simulink model "TosAeb"  (R2021a) (continued)
== system TosAeb/ELK_Objsel/Select Object Info2/If Action Subsystem1 ==
  Index : Inport
  Objs_all : Inport  Port=2
  Action Port : ActionPort  ActionPortLabel=else
  Selector : Selector  InputPortWidth=32  IndexOptions=Index vector (port)  Indices=[1 3]  OutputSizes=1
  TargetInfo : Outport
  wires:
  Selector/1 -> TargetInfo/1
  [Index] -> Selector/2
  [Objs_all] -> Selector/1
  Objs_all/1 -> [Objs_all]
  Index/1 -> [Index]

== system TosAeb/ELK_Objsel/Select Object Info2/If Action Subsystem2 ==
  Action Port : ActionPort  ActionPortLabel=if(u1 == 255)
  Bus Creator1 : BusCreator  Inputs=64  OutDataTypeStr=Bus: Object_Struct  NonVirtualBus=on
  Constant : Constant  Value=0  OutDataTypeStr=int64_t
  Constant{1..2,4,8,14..18,20..22,24..27,29..36,38..42,47,50..55,57,62,64..72,74} (48 alike) : Constant  Value=0  OutDataTypeStr=single
  Constant10 : Constant  Value=0  OutDataTypeStr=uint16_t
  Constant11 : Constant  Value=0  OutDataTypeStr=uint16_t
  Constant12 : Constant  Value=0  OutDataTypeStr=bool_t
  Constant{6..7,9,13,19,37,63,73} (8 alike) : Constant  Value=0  OutDataTypeStr=uint8_t
  Constant23 : Constant  Value=100  OutDataTypeStr=single
  Constant28 : Constant  Value=0  OutDataTypeStr=bool_t
  Constant3 : Constant  Value=0  OutDataTypeStr=uint16_t
  Constant5 : Constant  Value=0  OutDataTypeStr=int64_t
  Out : Outport  OutDataTypeStr=Bus:Object_Struct
  wires:
  Constant47/1 -> Bus Creator1/45  "objLengthStd"
  Constant42/1 -> Bus Creator1/44  "objHeight"
  Constant41/1 -> Bus Creator1/42  "objLength"
  Constant40/1 -> Bus Creator1/43  "objWidth"
  Constant39/1 -> Bus Creator1/41  "yawRateStd"
  Constant38/1 -> Bus Creator1/40  "yawRate"
  Constant31/1 -> Bus Creator1/35  "ayAbsStd"
  Constant33/1 -> Bus Creator1/33  "vyAbsStd"
  Constant34/1 -> Bus Creator1/34  "axAbsStd"
  Constant53/1 -> Bus Creator1/36  "yawAngle"
  Constant55/1 -> Bus Creator1/38  "azimuthAngle"
  Constant54/1 -> Bus Creator1/37  "yawAngleStd"
  Constant57/1 -> Bus Creator1/39  "azimuthAngleStd"
  Constant52/1 -> Bus Creator1/32  "vxAbsStd"
  Constant51/1 -> Bus Creator1/31  "ayAbs"
  Constant50/1 -> Bus Creator1/30  "axAbs"
  Constant71/1 -> Bus Creator1/28  "vxAbs"
  Constant72/1 -> Bus Creator1/29  "vyAbs"
  Constant68/1 -> Bus Creator1/27  "ayRelStd"
  Constant27/1 -> Bus Creator1/23  "ayRel"
  Constant32/1 -> Bus Creator1/26  "axRelStd"
  Constant30/1 -> Bus Creator1/25  "vyRelStd"
  Constant29/1 -> Bus Creator1/24  "vxRelStd"
  Constant67/1 -> Bus Creator1/22  "axRel"
  Constant66/1 -> Bus Creator1/21  "vyRel"
  Constant74/1 -> Bus Creator1/20  "vxRel"
  Constant73/1 -> Bus Creator1/19  "surfacePos"
  Constant62/1 -> Bus Creator1/15  "dyRef"
  Constant64/1 -> Bus Creator1/18  "dyRefSurface"
  Constant65/1 -> Bus Creator1/17  "dxRefSurface"
  Constant63/1 -> Bus Creator1/16  "refPosition"
  Bus Creator1/1 -> Out/1
  Constant26/1 -> Bus Creator1/14  "dxRef"
  Constant25/1 -> Bus Creator1/13  "dyCenterStd"
  Constant8/1 -> Bus Creator1/12  "dyCenter"
  Constant24/1 -> Bus Creator1/11  "dxCenterStd"
  Constant21/1 -> Bus Creator1/10  "dxCenter"
  Constant20/1 -> Bus Creator1/9  "objTypeProb"
  Constant6/1 -> Bus Creator1/8  "objType"
  Constant5/1 -> Bus Creator1/7  "fusionType"
  Constant4/1 -> Bus Creator1/6  "objLifeCycle"
  Constant70/1 -> Bus Creator1/5  "objIsobsProb"
  Constant69/1 -> Bus Creator1/4  "objExitProb"
  Constant28/1 -> Bus Creator1/3  "objValid"
  Constant3/1 -> Bus Creator1/2  "objID"
  Constant/1 -> Bus Creator1/1  "objTimeStamp"
  Constant1/1 -> Bus Creator1/46  "objWidthStd"
  Constant2/1 -> Bus Creator1/47  "objHeightStd"
  Constant7/1 -> Bus Creator1/48  "movingStatus"
  Constant9/1 -> Bus Creator1/49  "movingDirection"
  Constant10/1 -> Bus Creator1/50  "lightStatus"
  Constant11/1 -> Bus Creator1/51  "doorStatus"
  Constant12/1 -> Bus Creator1/52  "trailerStatus"
  Constant13/1 -> Bus Creator1/53  "laneAssign"
  Constant14/1 -> Bus Creator1/54  "laneAssociateConf"
  Constant15/1 -> Bus Creator1/55  "laneLeftPortion"
  Constant16/1 -> Bus Creator1/56  "laneRightPortion"
  Constant17/1 -> Bus Creator1/57  "laneLeftConf"
  Constant18/1 -> Bus Creator1/58  "laneRightConf"
  Constant19/1 -> Bus Creator1/59  "objIntention"
  Constant22/1 -> Bus Creator1/60  "objIntentionProb"
  Constant23/1 -> Bus Creator1/61  "objTTC"
  Constant35/1 -> Bus Creator1/62  "objLatDist2LaneCenter"
  Constant36/1 -> Bus Creator1/63  "objLatDist2EgoCenter"
  Constant37/1 -> Bus Creator1/64  "objPosition"

== system TosAeb/ELK_Objsel/Target_Selection/If Action Subsystem ==
  note: ELK Target Selection
  ForIterator_enum : Inport
  EPSILON : Inport  Port=2
  Objs_Obstacles : Inport  Port=3
  Host_Veh_Length : Inport  Port=4
  Host_Veh_Width : Inport  Port=5
  dxDiffObjCoord2VehCoord : Inport  Port=6
  VehSpdMps : Inport  Port=7
  YawRate_radps : Inport  Port=8
  YawRate_Degps : Inport  Port=9
  SteerAngle_Deg : Inport  Port=10
  SteerAngleSpd_degps : Inport  Port=11
  EgoTrajCurvature : Inport  Port=12
  EgoTrajCurvatureForFVSR : Inport  Port=13
  EgoTrajCurvature1 : Inport  Port=14
  EgoTrajCurvature2 : Inport  Port=15
  EgoTrajCurvature3 : Inport  Port=16
  EgoTrajCurvature4 : Inport  Port=17
  LDCoef_L : Inport  Port=18
  LDCoef_R : Inport  Port=19
  Action Port : ActionPort  ActionPortLabel=if(u1 ~= 0)
  Bus Selector : BusSelector  OutputSignals=LineWidth,LineA0,LineA1,LineA2,LineA3,LineStart,LineEnd
  Bus Selector1 : BusSelector  OutputSignals=dxCenter,dyCenter,vxRel,vyRel
  Bus Selector2 : BusSelector  OutputSignals=LineWidth,LineA0,LineA1,LineA2,LineA3,LineStart,LineEnd
  ELK_TargetSelection : Subsystem
  MATLAB Function : MATLAB Function  TreatAsAtomicUnit=on
  ObjCoord2VehCoord : Subsystem
  TTC_Calculation : ObjSelect_lib/TTC_Calculation  SystemSampleTime=-1  VariantActivationTime=update diagram  Latency=0  AutoFrameSizeCalculation=off
  Terminator{1..8} (8 alike) : Terminator
  Out7 : Outport
  Out8 : Outport  Port=2
  wires:
  MATLAB Function/3(vrAbs_f) -> [vrAbsObj]
  MATLAB Function/1(vxvAbs_f) -> [vxAbsObj]
  MATLAB Function/2(vyvAbs_f) -> [vyAbsObj]
  [ObjsSelect_LatDist] -> ObjCoord2VehCoord/2(dyObj)
  [dyObj_VehCoord] -> MATLAB Function/2(dyv_f)
  [dxDiffObjCoord2VehCoord] -> ObjCoord2VehCoord/3(dxDiffObjCoord2VehCoord)
  [dxObj_VehCoord] -> MATLAB Function/1(dxv_f)
  [ObjsSelect_LongiDist] -> ObjCoord2VehCoord/1(dxObj)
  [YawRate_radps] -> MATLAB Function/6(psiDtEgo_f)
  [ObjsSelect_LatVel] -> MATLAB Function/4(vyvRel_f)
  [VehSpdMps] -> MATLAB Function/5(vxvEgo_f)
  [ObjsSelect_LongiVel] -> MATLAB Function/3(vxvRel_f)
  ObjCoord2VehCoord/1(dxObj_VehCoord) -> [dxObj_VehCoord]
  ObjCoord2VehCoord/2(dyObj_VehCoord) -> [dyObj_VehCoord]
  Bus Selector1/2 -> [ObjsSelect_LatDist]  "<dyCenter>"
  Bus Selector1/4 -> [ObjsSelect_LatVel]  "<vyRel>"
  Bus Selector1/1 -> [ObjsSelect_LongiDist]  "<dxCenter>"
  Bus Selector1/3 -> [ObjsSelect_LongiVel]  "<vxRel>"
  [Objs_Obstacles] -> Bus Selector1/1
  ForIterator_enum/1 -> [ForIterator_enum]
  EPSILON/1 -> Terminator6/1
  Objs_Obstacles/1 -> [Objs_Obstacles]
  Host_Veh_Length/1 -> Terminator7/1
  Host_Veh_Width/1 -> [Host_Veh_Width]
  dxDiffObjCoord2VehCoord/1 -> [dxDiffObjCoord2VehCoord]
  VehSpdMps/1 -> [VehSpdMps]
  YawRate_radps/1 -> [YawRate_radps]
  YawRate_Degps/1 -> Terminator1/1
  SteerAngle_Deg/1 -> Terminator2/1
  SteerAngleSpd_degps/1 -> Terminator3/1
  EgoTrajCurvature/1 -> Terminator4/1
  LDCoef_L/1 -> [LDCoef_L]
  LDCoef_R/1 -> [LDCoef_R]
  [Objs_Obstacles] -> ELK_TargetSelection/2(Objs_all)
  [Host_Veh_Width] -> ELK_TargetSelection/3(Host_Veh_Width)
  [left_boundary_lat_rate_filtered] -> ELK_TargetSelection/4(left_boundary_lat_rate_filtered)
  [right_boundary_lat_rate_filtered] -> ELK_TargetSelection/5(right_boundary_lat_rate_filtered)
  [LDCoef_L] -> Bus Selector/1
  Bus Selector/2 -> ELK_TargetSelection/9(a0_LeftLine)  "<LineA0>"
  Bus Selector/1 -> ELK_TargetSelection/8(left_line_width)  "<LineWidth>"
  [LDCoef_R] -> Bus Selector2/1
  Bus Selector2/1 -> ELK_TargetSelection/15(right_line_width)  "<LineWidth>"
  Bus Selector2/2 -> ELK_TargetSelection/16(a0_RightLine)  "<LineA0>"
  Bus Selector/3 -> ELK_TargetSelection/10(a1_LeftLine)  "<LineA1>"
  Bus Selector/4 -> ELK_TargetSelection/11(a2_LeftLine)  "<LineA2>"
  Bus Selector/5 -> ELK_TargetSelection/12(a3_LeftLine)  "<LineA3>"
  Bus Selector/6 -> ELK_TargetSelection/13(SP_LeftLine)  "<LineStart>"
  Bus Selector/7 -> ELK_TargetSelection/14(EP_LeftLine)  "<LineEnd>"
  Bus Selector2/3 -> ELK_TargetSelection/17(a1_RightLine)  "<LineA1>"
  Bus Selector2/4 -> ELK_TargetSelection/18(a2_RightLine)  "<LineA2>"
  Bus Selector2/5 -> ELK_TargetSelection/19(a3_RightLine)  "<LineA3>"
  Bus Selector2/6 -> ELK_TargetSelection/20(SP_RightLine)  "<LineStart>"
  Bus Selector2/7 -> ELK_TargetSelection/21(EP_RightLine)  "<LineEnd>"
  [ELK_LeftLine_Valid_Flag] -> ELK_TargetSelection/6(ELK_LeftLine_Valid_Flag)
  [ELK_RightLine_Valid_Flag] -> ELK_TargetSelection/7(ELK_RightLine_Valid_Flag)
  [vxAbsObj] -> ELK_TargetSelection/22(vxAbsObj)
  [vyAbsObj] -> ELK_TargetSelection/23(vyAbsObj)
  [ForIterator_enum] -> ELK_TargetSelection/1(ForIterator_enum)
  ELK_TargetSelection/1(TA_Left_Flag) -> [TA_Left_Flag]
  ELK_TargetSelection/2(TA_Right_Flag) -> [TA_Right_Flag]
  [TTC_Calculated] -> ELK_TargetSelection/24(TTC_Calculated)
  [TA_Left_Flag] -> Out7/1
  [TA_Right_Flag] -> Out8/1
  EgoTrajCurvature1/1 -> [left_boundary_lat_rate_filtered]
  EgoTrajCurvature2/1 -> [right_boundary_lat_rate_filtered]
  EgoTrajCurvature3/1 -> [ELK_LeftLine_Valid_Flag]
  EgoTrajCurvature4/1 -> [ELK_RightLine_Valid_Flag]
  TTC_Calculation/1 -> [TTC_Calculated]
  [ObjsSelect_LongiDist] -> TTC_Calculation/1
  [ObjsSelect_LongiVel] -> TTC_Calculation/2
  EgoTrajCurvatureForFVSR/1 -> Terminator5/1
  [vrAbsObj] -> Terminator8/1

== system TosAeb/ELK_Objsel/Target_Selection/If Action Subsystem1 ==
  Action Port : ActionPort  ActionPortLabel=else
  Constant7 : Constant  Value=0  OutDataTypeStr=boolean
  Constant8 : Constant  Value=0  OutDataTypeStr=boolean
  Out7 : Outport
  Out8 : Outport  Port=2
  wires:
  Constant8/1 -> Out8/1
  Constant7/1 -> Out7/1

== system TosAeb/ELK_Objsel/Target_Selection/Subsystem ==
  Objs_Obstacles : Inport
  Bus Assignment : BusAssignment  AssignedSignals=objType,movingStatus
  Bus Selector : BusSelector  OutputSignals=objType,movingStatus,movingDirection,vyRel
  state_rebuild : Subsystem
  type_rebuild : Subsystem
  Obstacles : Outport
  wires:
  Objs_Obstacles/1 -> Bus Selector/1, Bus Assignment/1
  Bus Assignment/1 -> Obstacles/1
  Bus Selector/1 -> type_rebuild/1(Obj_type)  "<objType>"
  Bus Selector/2 -> state_rebuild/1(State)  "<movingStatus>"
  Bus Selector/3 -> state_rebuild/2(Orientation)  "<movingDirection>"
  type_rebuild/1(ObjType) -> Bus Assignment/2
  Bus Selector/4 -> state_rebuild/3(vy)  "<vyRel>"
  state_rebuild/1(obj_state) -> Bus Assignment/3

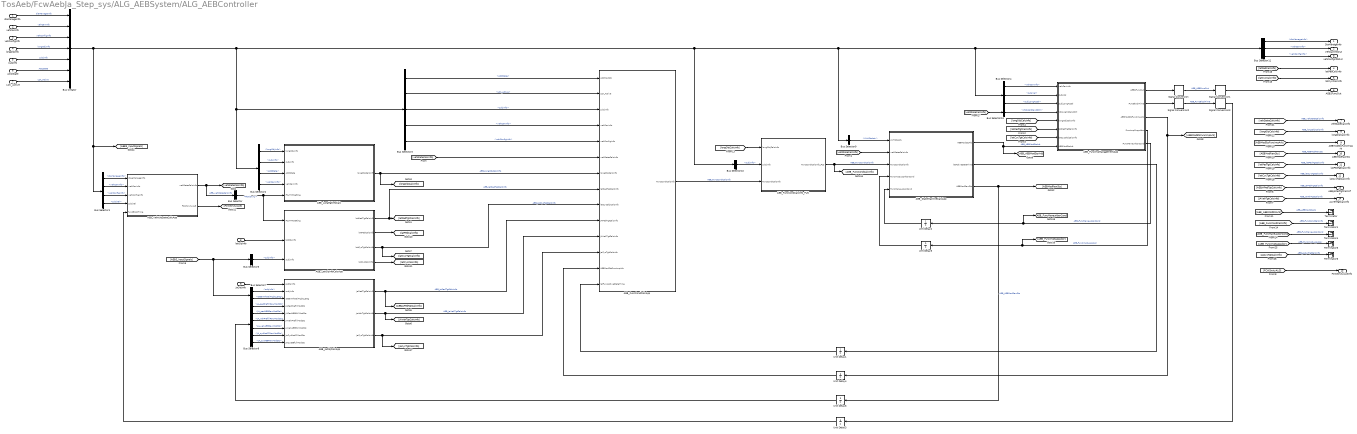
== system TosAeb/FcwAebJa_Step_sys/ALG_AEBSystem/ALG_AEBController ==
  diaManageInfo : Inport  OutDataTypeStr=Bus: BusDiagManageInfo
  vehCanInfo : Inport  Port=2  OutDataTypeStr=Bus: BusVehCanInfo
  vehConfigInfo : Inport  Port=3  OutDataTypeStr=Bus: BusVehConfigInfo
  longObjInfo : Inport  Port=4  OutDataTypeStr=Bus: BusAEBLongObj
  subjInfo : Inport  Port=5  OutDataTypeStr=Bus: BusSubjInfo
  ACCState : Inport  Port=6
  LCK_Active : Inport  Port=7
  latObjInfo : Inport  Port=8  OutDataTypeStr=Bus: BusAEBLatObj  PortDimensions=10  BusOutputAsStruct=on
  JaObjInfo : Inport  Port=9  OutDataTypeStr=Bus: BusAEBJAObjBus  PortDimensions=32  BusOutputAsStruct=on
  AEB_FuncCondCalcApp : Subsystem  TreatAsAtomicUnit=on
  AEB_FuncCondCalcInfo_FUS : Subsystem  TreatAsAtomicUnit=on
  AEB_FuncImpManagementApp : Subsystem  TreatAsAtomicUnit=on
  AEB_JaObjCalcApp : Subsystem  TreatAsAtomicUnit=on
  AEB_LatObjInfoCalcApp : Subsystem  TreatAsAtomicUnit=on
  AEB_LongTgtInfoCalc : Subsystem  TreatAsAtomicUnit=on
  AEB_StateMachineCalcApp : Subsystem  TreatAsAtomicUnit=on
  AEB_VehicleStateCalcApp : Subsystem  TreatAsAtomicUnit=on
  Bus Creator : BusCreator  Inputs=7
  Bus Selector : BusSelector  OutputSignals=VSC_AEBFuncSwtSts.FCWSnvty
  Bus Selector1 : BusSelector  OutputSignals=diaManageInfo,vehCanInfo,vehConfigInfo,subjInfo.subjVel
  Bus Selector10 : BusSelector  OutputSignals=subjInfo
  Bus Selector11 : BusSelector  OutputSignals=diaManageInfo,vehCanInfo,vehConfigInfo
  Bus Selector13 : BusSelector  OutputSignals=VSCVehStandStill
  Bus Selector2 : BusSelector  OutputSignals=longObjInfo,subjInfo,ACCState,vehCanInfo
  Bus Selector3 : BusSelector  OutputSignals=ACCState,LCK_Active,subjInfo,vehCanInfo,vehConfigInfo
  Bus Selector4 : BusSelector  OutputSignals=vehCanInfo,subjInfo.subjVel,subjInfo.subjLongAccel
  Bus Selector5 : BusSelector  OutputSignals=subjInfo
  Bus Selector7 : BusSelector  OutputSignals=subjInfo,vehCanInfo.SteerWheelAngle_Deg
  Bus Selector8 : BusSelector  OutputSignals=JA_AEBRawModSts.JA_pedPrefillRawModSts,JA_AEBRawModSts.JA_pedAEBRawModSts,JA_AEBRawModSts.JA_vehPrefillRawModSts,JA_AEBRawModSts.JA_vehAEBRawModSts,JA_AEBRawModSts.JA_cycPrefillRawModSts,JA_AEBRawModSts.JA_cycAEBRawModSts
  Bus Selector9 : BusSelector  OutputSignals=vehCanInfo.AVHStsActv
  Signal Conversion{,1..3} (4 alike) : SignalConversion  OverrideOpt=off
  Terminator{,1,4..6} (5 alike) : Terminator
  Unit Delay{1..6} (6 alike) : UnitDelay  SampleTime=-1  HasFrameUpgradeWarning=on
  DiaManageInfo : Outport  OutDataTypeStr=Bus: BusDiagManageInfo
  VehCanInfoOut : Outport  Port=2  OutDataTypeStr=Bus: BusVehCanInfo
  vehConfigInfoOut : Outport  Port=3  OutDataTypeStr=Bus: BusVehConfigInfo
  latPedCalcInfo : Outport  Port=4  OutDataTypeStr=Bus: BusLatPedObjCalcInfo
  latCycCalcInfo : Outport  Port=5  OutDataTypeStr=Bus: BusLatCycObjCalcInfo
  AEBSFuncOut : Outport  Port=6  OutDataTypeStr=Bus: BusAEBSFuncOutput
  vehStateCalcInfo : Outport  Port=7  OutDataTypeStr=Bus: BusVehStateCalcInfo
  longObjCalcInfo : Outport  Port=8  OutDataTypeStr=Bus: BusLongObjCalcInfo
  AEBModStsFuncImpArb : Outport  Port=9  OutDataTypeStr=Bus: BusAEBModStsFuncImpArb
  AEBModRawSts : Outport  Port=10  OutDataTypeStr=Bus: BusAEBModRawSts
  latPedTgtCalcInfo : Outport  Port=11  OutDataTypeStr=Bus: BusLatPedTgtCalcInfo
  latCycTgtCalcInfo : Outport  Port=12  OutDataTypeStr=Bus: BusLatCycTgtCalcInfo
  AEBJAPedTgtCalcInfo : Outport  Port=13  OutDataTypeStr=Bus: BusAEBJAPedTgtCalcInfo
  JAVehTgtCalcInfo : Outport  Port=14  OutDataTypeStr=Bus: BusAEBJAVehTgtCalcInfo
  FCWSnvtyALGInfo : Outport  Port=15
  wires:
  AEB_FuncImpManagementApp/1(AEBSFuncOut) -> Signal Conversion/1
  [JaCycTgtCalcInfo] -> Terminator6/1  "AEB_JaCycTgtCalcInfo"
  Bus Selector10/1 -> AEB_FuncCondCalcInfo_FUS/2(subjInfo)  "<subjInfo>"
  Bus Selector8/2 -> AEB_JaObjCalcApp/5(JAPedAEBfullModSts)  "<JA_pedAEBRawModSts>"
  Unit Delay5/1 -> AEB_StateMachineCalcApp/4(FuncImpSuppAborCond)
  [longObjCalcInfo] -> longObjCalcInfo/1  "AEB_longObjCalcInfo"
  AEB_JaObjCalcApp/3(JaCycTgtCalcInfo) -> AEB_FuncCondCalcApp/12(JaCycTgtCalcInfo), [JaCycTgtCalcInfo]
  [longObjCalcInfo] -> AEB_FuncImpManagementApp/5(longObjCalcInfo)
  Unit Delay6/1 -> Bus Selector8/1
  Bus Selector3/3 -> AEB_FuncCondCalcApp/3(subjInfo)  "<subjInfo>"
  Bus Selector8/5 -> AEB_JaObjCalcApp/8(JaCycPrefillModSts)  "<JA_cycPrefillRawModSts>"
  [vehStateCalcInfo] -> vehStateCalcInfo/1  "AEB_vehStateCalcInfo"
  [JAVehTgtCalcInfo] -> JAVehTgtCalcInfo/1  "AEB_JaVehTgtCalcInfo"
  Bus Selector8/6 -> AEB_JaObjCalcApp/9(JaCycEBfullModSts)  "<JA_cycAEBRawModSts>"
  [latCycCalcInfo] -> latCycCalcInfo/1
  AEB_FuncImpManagementApp/3(AEBModStsFuncImpArb) -> [AEBModStsFuncImpArb], Unit Delay4/1
  [vehStateCalcInfo] -> AEB_FuncCondCalcApp/6(vehStateCalcInfo)
  Bus Selector1/2 -> AEB_VehicleStateCalcApp/2(vehCanInfo)  "<vehCanInfo>"
  AEB_LatObjInfoCalcApp/1(latPedTgtCalcInfo) -> AEB_FuncCondCalcApp/8(latPedTgtCalcInfo), [latPedTgtCalcInfo]  "AEB_latPedTgtCalcInfo"
  [AEBS_InputSignals] -> Bus Selector5/1, Bus Selector7/1
  vehCanInfo/1 -> Bus Creator/2  "vehCanInfo"
  Unit Delay2/1 -> AEB_StateMachineCalcApp/5(FuncManeuAborCond)
  Bus Selector8/3 -> AEB_JaObjCalcApp/6(JAVehPrefillModSts)  "<JA_vehPrefillRawModSts>"
  AEB_FuncImpManagementApp/4(FuncImpSuppAbor) -> Unit Delay5/1, [AEB_FuncImpSuppAbor]  "AEB_FuncImpSuppAbor"
  AEB_StateMachineCalcApp/2(latActiveStateTime) -> Unit Delay1/1
  Bus Selector3/1 -> AEB_FuncCondCalcApp/1(ACCModSts)  "<ACCState>"
  AEB_JaObjCalcApp/1(JaPedTgtCalcInfo) -> AEB_FuncCondCalcApp/10(JAPedTgtCalcInfo), [AEBJAPedTgtCalcInfo]  "AEB_JaPedTgtCalcInfo"
  AEB_JaObjCalcApp/2(JaVehTgtCalcInfo) -> AEB_FuncCondCalcApp/11(JAVehTgtCalcInfo), [JAVehTgtCalcInfo]  "AEB_JaVehTgtCalcInfo"
  Bus Selector2/2 -> AEB_LongTgtInfoCalc/2(subjInfo)  "<subjInfo>"
  [latPedCalcInfo] -> latPedCalcInfo/1
  Bus Selector3/4 -> AEB_FuncCondCalcApp/4(vehCanInfo)  "<vehCanInfo>"
  Bus Selector2/3 -> AEB_LongTgtInfoCalc/3(ACCState)  "<ACCState>"
  [AEB_FuncCondCalcInfo] -> Terminator1/1  "AEB_FuncCondCalcInfo"
  AEB_FuncCondCalcInfo_FUS/1(FuncCondCalcInfo_FUS) -> [AEB_FuncCondCalcInfo], AEB_StateMachineCalcApp/3(FuncCondCalcInfo)  "AEB_FuncCondCalcInfo"
  latObjInfo/1 -> AEB_LatObjInfoCalcApp/2(latObjInfo)
  Bus Selector7/1 -> AEB_JaObjCalcApp/2(subjInfo)  "<subjInfo>"
  AEB_FuncCondCalcApp/1(FuncCondCalcInfo) -> AEB_FuncCondCalcInfo_FUS/3(FuncCondCalcInfo)  "AEB_FuncCondCalcInfo"
  Bus Selector4/1 -> AEB_FuncImpManagementApp/1(vehCanInfo)  "<vehCanInfo>"
  Bus Selector8/4 -> AEB_JaObjCalcApp/7(JAVehAEBfullModSts)  "<JA_vehAEBRawModSts>"
  Unit Delay3/1 -> AEB_VehicleStateCalcApp/5(funcBlockTime)
  [AEBModRawSts] -> AEBModRawSts/1  "AEB_AEBModRawSts"
  subjInfo/1 -> Bus Creator/5  "subjInfo"
  Bus Creator/1 -> [AEBS_InputSignals], Bus Selector1/1, Bus Selector11/1, Bus Selector4/1, Bus Selector9/1, Bus Selector10/1, Bus Selector3/1, Bus Selector2/1
  Bus Selector11/3 -> vehConfigInfoOut/1  "<vehConfigInfo>"
  [latPedTgtCalcInfo] -> AEB_FuncImpManagementApp/6(latPedTgtCalcInfo)
  Bus Selector3/2 -> AEB_FuncCondCalcApp/2(LCK_Active)  "<LCK_Active>"
  [vehStateCalcInfo] -> AEB_StateMachineCalcApp/2(vehStateCalcInfo)
  AEB_VehicleStateCalcApp/1(vehStateCalcInfo) -> Bus Selector/1, [vehStateCalcInfo]  "AEB_vehStateCalcInfo"
  JaObjInfo/1 -> AEB_JaObjCalcApp/1(JaObjInfo)
  [latCycTgtCalcInfo] -> latCycTgtCalcInfo/1  "AEB_latCycTgtCalcInfo"
  Bus Selector3/5 -> AEB_FuncCondCalcApp/5(vehConfigInfo)  "<vehConfigInfo>"
  AEB_StateMachineCalcApp/3(AEBModRawSts) -> [AEBModRawSts], Unit Delay6/1  "AEB_AEBModRawSts"
  Bus Selector11/1 -> DiaManageInfo/1  "<diaManageInfo>"
  Bus Selector4/3 -> AEB_FuncImpManagementApp/3(subjLongAccel)  "<subjLongAccel>"
  [AEB_FuncManeuAborCond] -> Terminator4/1  "AEB_FuncManeuAborCond"
  Bus Selector2/1 -> AEB_LongTgtInfoCalc/1(longObjInfo)  "<longObjInfo>"
  [AEBModStsFuncImpArb] -> AEBModStsFuncImpArb/1
  AEB_StateMachineCalcApp/1(AEBModStsArb) -> [AEB_AEBModStsArb], AEB_FuncImpManagementApp/8(AEBModStsArb)  "AEB_AEBModStsArb"
  Signal Conversion2/1 -> Signal Conversion3/1  "AEB_FuncBlockTime"
  [latPedTgtCalcInfo] -> latPedTgtCalcInfo/1  "AEB_latPedTgtCalcInfo"
  Bus Selector2/4 -> AEB_LongTgtInfoCalc/4(vehCanInfo)  "<vehCanInfo>"
  Bus Selector9/1 -> AEB_StateMachineCalcApp/1(AVHStsActv)  "<AVHStsActv>"
  Bus Selector1/4 -> AEB_VehicleStateCalcApp/4(subjVel)  "<subjVel>"
  Bus Selector11/2 -> VehCanInfoOut/1  "<vehCanInfo>"
  Bus Selector1/1 -> AEB_VehicleStateCalcApp/1(diagManageInfo)  "<diaManageInfo>"
  Unit Delay1/1 -> AEB_FuncCondCalcApp/14(latFuncActiveStateTime)
  Signal Conversion/1 -> Signal Conversion1/1  "AEB_AEBFuncOut"
  Bus Selector4/2 -> AEB_FuncImpManagementApp/2(subjVel)  "<subjVel>"
  AEB_LatObjInfoCalcApp/4(latCycCalcInfo) -> [latCycCalcInfo]
  [AEB_FuncImpSuppAbor] -> Terminator5/1  "AEB_FuncImpSuppAbor"
  [AEB_AEBModStsArb] -> Terminator/1  "AEB_AEBModStsArb"
  Unit Delay4/1 -> AEB_FuncCondCalcApp/13(AEBModStsFuncImpArb)
  Signal Conversion1/1 -> AEBSFuncOut/1
  [AEBJAPedTgtCalcInfo] -> AEBJAPedTgtCalcInfo/1  "AEB_JaPedTgtCalcInfo"
  diaManageInfo/1 -> Bus Creator/1  "diaManageInfo"
  Bus Selector13/1 -> AEB_FuncImpManagementApp/4(VSC_VehStandstill)  "<VSCVehStandStill>"
  LCK_Active/1 -> Bus Creator/7  "LCK_Active"
  Signal Conversion3/1 -> Unit Delay3/1
  longObjInfo/1 -> Bus Creator/4  "longObjInfo"
  AEB_FuncImpManagementApp/2(FuncBlockTime) -> Signal Conversion2/1
  Bus Selector1/3 -> AEB_VehicleStateCalcApp/3(vehConfigInfo)  "<vehConfigInfo>"
  [longObjCalcInfo] -> AEB_FuncCondCalcInfo_FUS/1(longObjCalcInfo)
  ACCState/1 -> Bus Creator/6  "ACCState"
  Bus Selector/1 -> AEB_LatObjInfoCalcApp/1(FcwHMISetting), AEB_LongTgtInfoCalc/5(FcwHMISetting)  "<FCWSnvty>"
  AEB_LatObjInfoCalcApp/3(latCycTgtCalcInfo) -> AEB_FuncCondCalcApp/9(latCycObjCalcInfo), [latCycTgtCalcInfo]  "AEB_latCycTgtCalcInfo"
  AEB_LongTgtInfoCalc/1(longObjCalcInfo) -> AEB_FuncCondCalcApp/7(longObjCalcInfo), [longObjCalcInfo]  "AEB_longObjCalcInfo"
  Bus Selector7/2 -> AEB_JaObjCalcApp/3(SteerWheelAngle_Deg)  "<SteerWheelAngle_Deg>"
  Bus Selector8/1 -> AEB_JaObjCalcApp/4(JAPedPrefillModSts)  "<JA_pedPrefillRawModSts>"
  AEB_LatObjInfoCalcApp/2(latPedCalcInfo) -> [latPedCalcInfo]
  [latCycTgtCalcInfo] -> AEB_FuncImpManagementApp/7(latCycObjCalcInfo)
  vehConfigInfo/1 -> Bus Creator/3  "vehConfigInfo"
  Bus Selector5/1 -> AEB_LatObjInfoCalcApp/3(subjInfo)  "<subjInfo>"
  [vehStateCalcInfo] -> Bus Selector13/1
  AEB_FuncImpManagementApp/5(FuncManeuAborCond) -> Unit Delay2/1, [AEB_FuncManeuAborCond]  "AEB_FuncManeuAborCond"
  AEB_VehicleStateCalcApp/2(FCWSnvtyALG) -> [FCWSnvtyALG]
  [FCWSnvtyALG] -> FCWSnvtyALGInfo/1

== system TosAeb/FcwAebJa_Step_sys/ALG_AEBSystem/ALG_AEBInput ==
  TOS_AEB_LONOBJ : Inport  OutDataTypeStr=Bus: Object_Struct
  TOS_AEB_LATOBJ : Inport  Port=2  OutDataTypeStr=Bus: Object_Struct
  TOS_AEB_JAOBJ : Inport  Port=3  OutDataTypeStr=Bus: Object_Struct
  vxvRefMs : Inport  Port=4  OutDataTypeStr=single
  axvRefMs2 : Inport  Port=5  OutDataTypeStr=single
  ayvRefMs2 : Inport  Port=6  OutDataTypeStr=single
  kapTraj : Inport  Port=7  OutDataTypeStr=single
  psiDtOpt : Inport  Port=8  OutDataTypeStr=single
  ALG_VCAN_MSG : Inport  Port=9  OutDataTypeStr=Bus: VehicleInfo_Struct  PortDimensions=1  BusOutputAsStruct=on
  ADASStateMap : Subsystem  TreatAsAtomicUnit=on
  Bus Selector1 : BusSelector  OutputSignals=chasssisInfo.vehicleSpd
  Constant : Constant  Value=20240702  OutDataTypeStr=uint32
  DiaManageInfoMap : Subsystem  TreatAsAtomicUnit=on
  JaObjInfoMap : Subsystem  TreatAsAtomicUnit=on
  LatObjInfoMap : Subsystem  TreatAsAtomicUnit=on
  LongObjInfoMap : Subsystem  TreatAsAtomicUnit=on
  Terminator : Terminator
  VehCanInfoMap : Subsystem  TreatAsAtomicUnit=on
  VehConfigInfoMap : Subsystem  TreatAsAtomicUnit=on
  subjInfoMap : Subsystem  TreatAsAtomicUnit=on
  vxvRefMsFilt : Subsystem  TreatAsAtomicUnit=on
  diaManageInfo : Outport  OutDataTypeStr=Bus: BusDiagManageInfo
  vehCanInfo : Outport  Port=2
  vehConfigInfo : Outport  Port=3  OutDataTypeStr=Bus: BusVehConfigInfo
  longObjInfo : Outport  Port=4  OutDataTypeStr=Bus: BusAEBLongObj
  subjInfo : Outport  Port=5  OutDataTypeStr=Bus: BusSubjInfo
  ACCState : Outport  Port=6
  LCK_Active : Outport  Port=7
  latObjInfo : Outport  Port=8  OutDataTypeStr=Bus: BusAEBLatObj
  JaObjInfo : Outport  Port=9  OutDataTypeStr=Bus: BusAEBJAObjBus
  wires:
  TOS_AEB_LATOBJ/1 -> LatObjInfoMap/1(TOS_AEB_LATOBJ)
  psiDtOpt/1 -> subjInfoMap/5(psiDtOpt)
  VehCanInfoMap/1(vehCanInfo) -> vehCanInfo/1
  Constant/1 -> Terminator/1  "AEB_INPUT_VERSION"
  ayvRefMs2/1 -> subjInfoMap/3(ayvRefMs2)
  kapTraj/1 -> subjInfoMap/4(kapTraj)
  ADASStateMap/1(ACCState) -> ACCState/1
  TOS_AEB_JAOBJ/1 -> JaObjInfoMap/1(TOS_AEB_JAOBJ)
  vxvRefMs/1 -> vxvRefMsFilt/1(vxvRefMs)
  LongObjInfoMap/1(longObjInfo) -> longObjInfo/1
  ADASStateMap/2(LCK_Active) -> LCK_Active/1
  vxvRefMsFilt/1(vxvRefMsFilt) -> subjInfoMap/1(vxvRefMsFilt)
  DiaManageInfoMap/1(diaManageInfo) -> diaManageInfo/1
  JaObjInfoMap/1(JaObjInfo) -> JaObjInfo/1
  LatObjInfoMap/1(latObjInfo) -> latObjInfo/1
  axvRefMs2/1 -> subjInfoMap/2(axvRefMs2)
  subjInfoMap/1(subjInfo) -> subjInfo/1
  TOS_AEB_LONOBJ/1 -> LongObjInfoMap/1(TOS_AEB_LONOBJ)
  VehConfigInfoMap/1(vehConfigInfo) -> vehConfigInfo/1
  ALG_VCAN_MSG/1 -> Bus Selector1/1, VehCanInfoMap/1(ALG_VCAN_MSG)
  Bus Selector1/1 -> vxvRefMsFilt/2(VehSpd)  "<vehicleSpd>"
